simulink model "vegas01_4tap_1024ch_220_r8b"  (Simulink 7.6)
settings: Solver=ode45  StartTime=0.0  StopTime=128000  RelTol=1e-3
libraries: xbsIndex_r4 x12586, monroe_library x80, xbsReplacements_r4 x42, xps_library x34, casper_library_misc x27, dspsigops x16, simulink x7
(part 9 of 91)

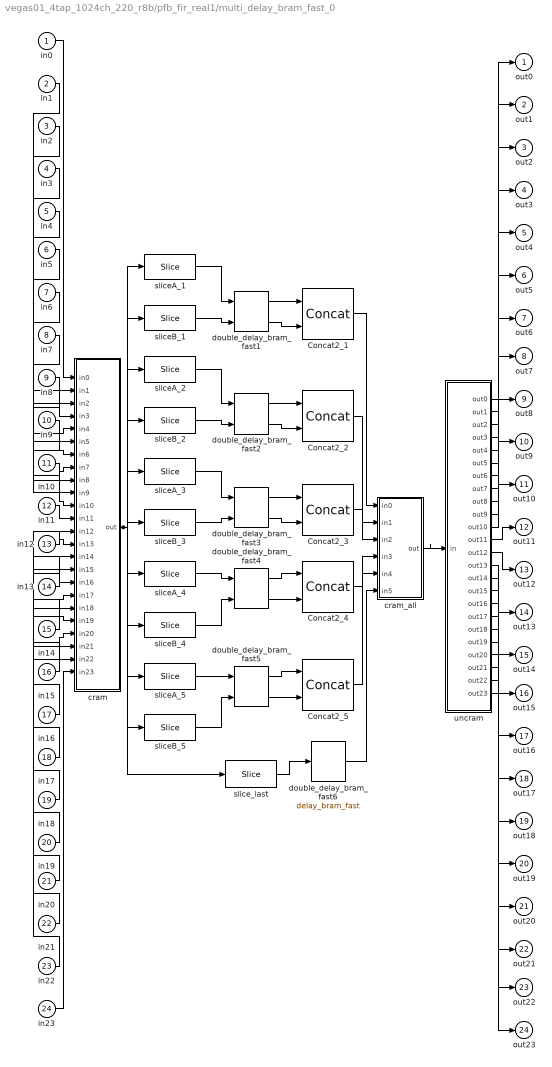
== system vegas01_4tap_1024ch_220_r8b/pfb_fir_real1/multi_delay_bram_fast_0 ==
  in0 : Inport
  in1 : Inport  Port=2
  in2 : Inport  Port=3
  in3 : Inport  Port=4
  in4 : Inport  Port=5
  in5 : Inport  Port=6
  in6 : Inport  Port=7
  in7 : Inport  Port=8
  in8 : Inport  Port=9
  in9 : Inport  Port=10
  in10 : Inport  Port=11
  in11 : Inport  Port=12
  in12 : Inport  Port=13
  in13 : Inport  Port=14
  in14 : Inport  Port=15
  in15 : Inport  Port=16
  in16 : Inport  Port=17
  in17 : Inport  Port=18
  in18 : Inport  Port=19
  in19 : Inport  Port=20
  in20 : Inport  Port=21
  in21 : Inport  Port=22
  in22 : Inport  Port=23
  in23 : Inport  Port=24
  Concat2_{1..5} (5 alike) : xbsIndex_r4/Concat  num_inputs=2  dbl_ovrd=off  block_type=concat
  cram : Subsystem
  cram_all : Subsystem
  double_delay_bram_fast{1..5} (5 alike) : monroe_library/double_delay_bram_fast  SystemSampleTime=-1  delay_len=256  bram_latency=2  register_counter=1
  double_delay_bram_fast6 : monroe_library/delay_bram_fast  SystemSampleTime=-1  delay_len=256  bram_latency=2  memory_type=Block RAM  register_counter=1
  sliceA_1 : xbsIndex_r4/Slice  nbits=18  boolean_output=off  mode=Upper Bit Location + Width  bit1=-0  base1=MSB of Input  bit0=-0  base0=MSB of Input  dbl_ovrd=off  block_type=slice
  sliceA_2 : xbsIndex_r4/Slice  nbits=18  boolean_output=off  mode=Upper Bit Location + Width  bit1=-36  base1=MSB of Input  bit0=-36  base0=MSB of Input  dbl_ovrd=off  block_type=slice
  sliceA_3 : xbsIndex_r4/Slice  nbits=18  boolean_output=off  mode=Upper Bit Location + Width  bit1=-72  base1=MSB of Input  bit0=-72  base0=MSB of Input  dbl_ovrd=off  block_type=slice
  sliceA_4 : xbsIndex_r4/Slice  nbits=18  boolean_output=off  mode=Upper Bit Location + Width  bit1=-108  base1=MSB of Input  bit0=-108  base0=MSB of Input  dbl_ovrd=off  block_type=slice
  sliceA_5 : xbsIndex_r4/Slice  nbits=18  boolean_output=off  mode=Upper Bit Location + Width  bit1=-144  base1=MSB of Input  bit0=-144  base0=MSB of Input  dbl_ovrd=off  block_type=slice
  sliceB_1 : xbsIndex_r4/Slice  nbits=18  boolean_output=off  mode=Upper Bit Location + Width  bit1=-18  base1=MSB of Input  bit0=-18  base0=MSB of Input  dbl_ovrd=off  block_type=slice
  sliceB_2 : xbsIndex_r4/Slice  nbits=18  boolean_output=off  mode=Upper Bit Location + Width  bit1=-54  base1=MSB of Input  bit0=-54  base0=MSB of Input  dbl_ovrd=off  block_type=slice
  sliceB_3 : xbsIndex_r4/Slice  nbits=18  boolean_output=off  mode=Upper Bit Location + Width  bit1=-90  base1=MSB of Input  bit0=-90  base0=MSB of Input  dbl_ovrd=off  block_type=slice
  sliceB_4 : xbsIndex_r4/Slice  nbits=18  boolean_output=off  mode=Upper Bit Location + Width  bit1=-126  base1=MSB of Input  bit0=-126  base0=MSB of Input  dbl_ovrd=off  block_type=slice
  sliceB_5 : xbsIndex_r4/Slice  nbits=18  boolean_output=off  mode=Upper Bit Location + Width  bit1=-162  base1=MSB of Input  bit0=-162  base0=MSB of Input  dbl_ovrd=off  block_type=slice
  slice_last : xbsIndex_r4/Slice  nbits=12  boolean_output=off  mode=Lower Bit Location + Width  bit1=0  base1=LSB of Input  bit0=0  base0=LSB of Input  dbl_ovrd=off  block_type=slice
  uncram : Subsystem
  out0 : Outport
  out1 : Outport  Port=2
  out2 : Outport  Port=3
  out3 : Outport  Port=4
  out4 : Outport  Port=5
  out5 : Outport  Port=6
  out6 : Outport  Port=7
  out7 : Outport  Port=8
  out8 : Outport  Port=9
  out9 : Outport  Port=10
  out10 : Outport  Port=11
  out11 : Outport  Port=12
  out12 : Outport  Port=13
  out13 : Outport  Port=14
  out14 : Outport  Port=15
  out15 : Outport  Port=16
  out16 : Outport  Port=17
  out17 : Outport  Port=18
  out18 : Outport  Port=19
  out19 : Outport  Port=20
  out20 : Outport  Port=21
  out21 : Outport  Port=22
  out22 : Outport  Port=23
  out23 : Outport  Port=24
  wires:
  cram/1(out) -> slice_last/1, sliceB_5/1, sliceA_5/1, sliceB_4/1, sliceA_4/1, sliceB_3/1, sliceA_3/1, sliceB_2/1, sliceA_2/1, sliceB_1/1, sliceA_1/1
  uncram/24(out23) -> out23/1
  uncram/23(out22) -> out22/1
  uncram/22(out21) -> out21/1
  uncram/21(out20) -> out20/1
  uncram/20(out19) -> out19/1
  uncram/19(out18) -> out18/1
  uncram/18(out17) -> out17/1
  uncram/17(out16) -> out16/1
  uncram/16(out15) -> out15/1
  uncram/15(out14) -> out14/1
  uncram/14(out13) -> out13/1
  uncram/13(out12) -> out12/1
  uncram/12(out11) -> out11/1
  uncram/11(out10) -> out10/1
  uncram/10(out9) -> out9/1
  uncram/9(out8) -> out8/1
  uncram/8(out7) -> out7/1
  uncram/7(out6) -> out6/1
  uncram/6(out5) -> out5/1
  uncram/5(out4) -> out4/1
  uncram/4(out3) -> out3/1
  uncram/3(out2) -> out2/1
  uncram/2(out1) -> out1/1
  uncram/1(out0) -> out0/1
  cram_all/1(out) -> uncram/1(in)
  double_delay_bram_fast6/1 -> cram_all/6(in5)
  Concat2_5/1 -> cram_all/5(in4)
  Concat2_4/1 -> cram_all/4(in3)
  Concat2_3/1 -> cram_all/3(in2)
  Concat2_2/1 -> cram_all/2(in1)
  Concat2_1/1 -> cram_all/1(in0)
  slice_last/1 -> double_delay_bram_fast6/1
  double_delay_bram_fast5/2 -> Concat2_5/2
  double_delay_bram_fast5/1 -> Concat2_5/1
  sliceB_5/1 -> double_delay_bram_fast5/2
  sliceA_5/1 -> double_delay_bram_fast5/1
  double_delay_bram_fast4/2 -> Concat2_4/2
  double_delay_bram_fast4/1 -> Concat2_4/1
  sliceB_4/1 -> double_delay_bram_fast4/2
  sliceA_4/1 -> double_delay_bram_fast4/1
  double_delay_bram_fast3/2 -> Concat2_3/2
  double_delay_bram_fast3/1 -> Concat2_3/1
  sliceB_3/1 -> double_delay_bram_fast3/2
  sliceA_3/1 -> double_delay_bram_fast3/1
  double_delay_bram_fast2/2 -> Concat2_2/2
  double_delay_bram_fast2/1 -> Concat2_2/1
  sliceB_2/1 -> double_delay_bram_fast2/2
  sliceA_2/1 -> double_delay_bram_fast2/1
  double_delay_bram_fast1/2 -> Concat2_1/2
  double_delay_bram_fast1/1 -> Concat2_1/1
  sliceB_1/1 -> double_delay_bram_fast1/2
  sliceA_1/1 -> double_delay_bram_fast1/1
  in23/1 -> cram/24(in23)
  in22/1 -> cram/23(in22)
  in21/1 -> cram/22(in21)
  in20/1 -> cram/21(in20)
  in19/1 -> cram/20(in19)
  in18/1 -> cram/19(in18)
  in17/1 -> cram/18(in17)
  in16/1 -> cram/17(in16)
  in15/1 -> cram/16(in15)
  in14/1 -> cram/15(in14)
  in13/1 -> cram/14(in13)
  in12/1 -> cram/13(in12)
  in11/1 -> cram/12(in11)
  in10/1 -> cram/11(in10)
  in9/1 -> cram/10(in9)
  in8/1 -> cram/9(in8)
  in7/1 -> cram/8(in7)
  in6/1 -> cram/7(in6)
  in5/1 -> cram/6(in5)
  in4/1 -> cram/5(in4)
  in3/1 -> cram/4(in3)
  in2/1 -> cram/3(in2)
  in1/1 -> cram/2(in1)
  in0/1 -> cram/1(in0)

== system vegas01_4tap_1024ch_220_r8b/pfb_fir_real3/coeff_gen_0_7 ==
  sync : Inport
  Counter0 : xbsIndex_r4/Counter  cnt_type=Free Running  cnt_to=Inf  operation=Up  start_count=8  cnt_by_val=1  arith_type=Unsigned  n_bits=8  bin_pt=0  load_pin=off  rst=on  en=off  explicit_period=on  period=1  dbl_ovrd=off  use_behavioral_HDL=off  implementation=Fabric  block_type=counter
  Counter1 : xbsIndex_r4/Counter  cnt_type=Free Running  cnt_to=Inf  operation=Down  start_count=247  cnt_by_val=1  arith_type=Unsigned  n_bits=8  bin_pt=0  load_pin=off  rst=on  en=off  explicit_period=on  period=1  dbl_ovrd=off  use_behavioral_HDL=off  implementation=Fabric  block_type=counter
  Delay1 : xbsIndex_r4/Delay  rst=off  infoeditControl=Selection of Reset will increase slice count due to use of real FFs and instead of SRLs  en=off  latency=2  dbl_ovrd=off  reg_retiming=off  block_type=delay
  Delay2 : xbsIndex_r4/Delay  rst=off  infoeditControl=Selection of Reset will increase slice count due to use of real FFs and instead of SRLs  en=off  latency=1  dbl_ovrd=off  reg_retiming=off  block_type=delay
  Delay3 : xbsIndex_r4/Delay  rst=off  infoeditControl=Selection of Reset will increase slice count due to use of real FFs and instead of SRLs  en=off  latency=1  dbl_ovrd=off  reg_retiming=off  block_type=delay
  Delay4 : xbsIndex_r4/Delay  rst=off  infoeditControl=Selection of Reset will increase slice count due to use of real FFs and instead of SRLs  en=off  latency=2  dbl_ovrd=off  reg_retiming=off  block_type=delay
  coeff_ram_1 : xbsIndex_r4/Dual Port RAM  depth=256  initVector=[-9.76681628083641e-06 -0.000166374153578663 -0.000323674631039594 -0.000481750704462836 -0.000640685368821483 -0.000800562109341241 -0.000961464852068701 -0.00112347791394463 -0.00128668595239865 -0.00145117391448125 -0.00161702698554985 -0.00178433053752527 -0.00195317007673584 -0.00212363119136582 -0.00229579949852553 -0.00246976059096078 -0.00264559998341877 -0.00282340305868871 -0.00300325501333463 -0.003185240803139 -0.00336944508827481 -0.00355595217822514 -0.00374484597646818 -0.00393620992494696 -0.0041301269483421 -0.00432667939816694 -0.00452594899670393 -0.00472801678080149 -0.00493296304555074 -0.00514086728786142 -0.00535180814995662 -0.0055658633628058 -0.00578310968951604 -0.00600362286870091 -0.00622747755784734 -0.00645474727669994 -0.00668550435068305 -0.00691981985438059 -0.00715776355509352 -0.00739940385649554 -0.00764480774240668 -0.00789404072070559 -0.00814716676740016 -0.00840424827087738 -0.00866534597635216 -0.00893051893053586 -0.00919982442654445 -0.00947331794906695 -0.00975105311981421 -0.0100330816432682 -0.0103194532527527 -0.010610215656844 -0.0109054144861447 -0.0112050932404367 -0.011509293236238 -0.0118180535547789 -0.0121314109904214 -0.0124493999995389 -0.0127720526498779 -0.0130993985704197 -0.0134314649017638 -0.0137682762470507 -0.0141098546234445 -0.0144562194141947 -0.0148073873212959 -0.0151633723187648 -0.015524185606554 -0.0158898355651202 -0.0162603277106669 -0.0166356646510793 -0.0170158460425702 -0.0174008685470547 -0.0177907257902727 -0.0181854083206757 -0.0185849035690979 -0.0189891958092265 -0.0193982661188903 -0.0198120923421836 -0.0202306490524425 -0.0206539075160887 -0.0210818356573608 -0.0215143980239454 -0.0219515557535275 -0.0223932665412744 -0.0228394846082689 -0.0232901606709079 -0.0237452419112808 -0.0242046719485435 -0.0246683908113017 -0.0251363349110189 -0.0256084370164626 -0.0260846262292031 -0.0265648279601778 -0.0270489639073352 -0.0275369520343712 -0.0280287065505702 -0.0285241378917642 -0.0290231527024214 -0.0295256538188765 -0.0300315402537142 -0.0305407071813169 -0.0310530459245885 -0.0315684439428629 -0.0320867848210099 -0.0326079482597467 -0.0331318100671657 -0.0336582421514863 -0.0341871125150421 -0.0347182852495099 -0.0352516205323896 -0.0357869746247431 -0.0363241998701992 -0.0368631446952324 -0.0374036536107213 -0.0379455672147943 -0.0384887221969679 -0.0390329513435835 -0.0395780835445478 -0.0401239438013823 -0.0406703532365864 -0.0412171291043174 -0.0417640848023921 -0.0423110298856138 -0.0428577700804262 -0.0434041073008984 -0.0439498396660427 -0.0444947615184662 -0.0450386634443597 -0.0455813322948223 -0.0461225512085252 -0.0466620996357123 -0.0471997533635396 -0.0477352845427513 -0.0482684617156931 -0.0487990498456597 -0.0493268103475761 -0.0498515011200101 -0.0503728765785135 -0.0508906876902881 -0.0514046820101748 -0.0519146037179613 -0.0524201936570026 -0.0529211893741533 -0.0534173251610023 -0.0539083320964084 -0.0543939380903286 -0.0548738679289332 -0.0553478433210022 -0.0558155829455944 -0.0562768025009831 -0.0567312147548497 -0.0571785295957271 -0.0576184540856848 -0.0580506925142456 -0.0584749464535256 -0.0588909148145869 -0.0592982939049929 -0.0596967774875561 -0.0600860568402664 -0.0604658208173894 -0.0608357559117222 -0.0611955463179954 -0.0615448739974078 -0.0618834187432817 -0.0622108582478242 -0.062526868169983 -0.0628311222043803 -0.0631232921513118 -0.0634030479877959 -0.0636700579396572 -0.0639239885546298 -0.0641645047764638 -0.0643912700200192 -0.0646039462473303 -0.0648021940446247 -0.0649856727002773 -0.0651540402836856 -0.065306953725044 -0.0654440688960025 -0.0655650406911897 -0.06566952311058 -0.0657571693426894 -0.0658276318485765 -0.0658805624466314 -0.0659156123981315 -0.0659324324935443 -0.0659306731395561 -0.0659099844468056 -0.0658700163183022 -0.0658104185385061 -0.0657308408630492 -0.065630933109075 -0.0655103452461749 -0.0653687274878981 -0.0652057303838135 -0.0650210049120985 -0.0648142025726343 ...(+1180 chars)]  distributed_mem=Block RAM  init_a=0  init_b=0  rst_a=off  rst_b=off  en_a=off  en_b=off  latency=2  write_mode_A=No Read On Write  write_mode_B=No Read On Write  dbl_ovrd=off  optimize=Area  use_rpm=on  block_type=dpram
  coeff_ram_2 : xbsIndex_r4/Dual Port RAM  depth=256  initVector=[0.000131889660979235 0.0022626199016281 0.00443201888730544 0.00664021076774132 0.00888731333319452 0.011173437943922 0.013498689460842 0.0158631661774126 0.0182669597527502 0.0207101551460066 0.0231928305520307 0.0257150573383326 0.0282768999833737 0.0308784160162031 0.0335196559574605 0.0362006632617669 0.0389214742615229 0.041682118112133 0.0444826167386781 0.0473229847840524 0.0502032295585852 0.0531233509911659 0.0560833415818892 0.0590831863562395 0.0621228628208312 0.0652023409207219 0.0683215829983153 0.07148054375387 0.0746791702076299 0.0779174016635925 0.0811951696749281 0.084512398011068 0.0878690026264726 0.0912648916310948 0.0946999652625533 0.0981741158600261 0.10168722783988 0.105239177673046 0.108829833864151 0.112459056932427 0.116126699394389 0.119832605748316 0.123576612460526 0.127358547953464 0.131178232595614 0.135035478693229 0.138930090483912 0.142861864132034 0.146830587726002 0.150836041277392 0.154877996721945 0.158956217922433 0.163070460673406 0.167220472707813 0.171405993705518 0.175626755303703 0.179882481109162 0.184172886712494 0.188497679704198 0.192856559692668 0.197249218324085 0.201675339304225 0.206134598422161 0.210626663575877 0.215151194799783 0.219707844294133 0.224296256456348 0.228916067914241 0.233566907561132 0.238248396592867 0.242960148546725 0.247701769342212 0.252472857323742 0.257273003305192 0.262101790616336 0.266958795151142 0.271843585417932 0.276755722591397 0.28169476056646 0.286660246013975 0.291651718438258 0.296668710236444 0.301710746759654 0.306777346375963 0.311868020535164 0.316982273835315 0.322119604091054 0.327279502403677 0.332461453232956 0.337664934470704 0.342889417516048 0.348134367352417 0.353399242626224 0.358683495727226 0.363986572870548 0.369307914180353 0.374646953775156 0.380003119854737 0.385375834788674 0.390764515206442 0.396168572089086 0.401587410862443 0.407020431491882 0.412467028578566 0.417926591457195 0.423398504295229 0.428882146193547 0.434376891288555 0.439882108855684 0.445397163414288 0.450921414833902 0.456454218441849 0.461994925132158 0.467542881475792 0.473097429832141 0.478657908461773 0.48422365164041 0.489793989774102 0.495368249515587 0.500945753881801 0.506525822372513 0.512107771090063 0.517690912860181 0.523274557353848 0.528858011210183 0.534440578160332 0.540021559152312 0.545600252476811 0.551175953893889 0.556747956760575 0.562315552159312 0.567878029027235 0.573434674286248 0.578984772973868 0.584527608374814 0.590062462153306 0.595588614486041 0.601105344195828 0.606611928885839 0.61210764507445 0.617591768330647 0.623063573409952 0.628522334390862 0.633967324811734 0.639397817808129 0.644813086250539 0.650212402882503 0.655595040459053 0.660960271885469 0.666307370356321 0.671635609494744 0.676944263491929 0.682232607246797 0.687499916505816 0.692745468002935 0.697968539599602 0.703168410424828 0.708344361015273 0.713495673455308 0.718621631517039 0.723721520800239 0.728794628872177 0.733840245407289 0.738857662326676 0.743846173937386 0.748805077071453 0.753733671224655 0.758631258694962 0.763497144720641 0.768330637617979 0.773131048918607 0.77789769350637 0.782629889753732 0.787326959657669 0.791988228975025 0.796613027357295 0.801200688484807 0.805750550200266 0.810261954641631 0.814734248374296 0.819166782522532 0.823558912900179 0.827910000140534 0.832219409825417 0.836486512613386 0.840710684367061 0.844891306279528 0.849027764999809 0.853119452757334 0.857165767485426 0.861166112943733 0.865119898839605 0.869026540948364 0.872885461232459 0.876696087959463 0.880457855818883 0.884170206037768 0.887832586495072 0.891444451834754 0.895005263577584 0.898514490231627 0.90197160740138 0.905376097895536 0.908727451833345 0.912025166749558 0.915268747697902 0.9184577073531 0.921591566111373 0.924669852189424 0.927692101721869 0.930657858857099 0.933566675851536 0.936418113162282 0.939211739538114 0.941947132108819 0.944623876472843 0.947241566783232 0.949799805831843 0.952298205131806 0.954736384998219 ...(+644 chars)]  distributed_mem=Block RAM  init_a=0  init_b=0  rst_a=off  rst_b=off  en_a=off  en_b=off  latency=2  write_mode_A=No Read On Write  write_mode_B=No Read On Write  dbl_ovrd=off  optimize=Area  use_rpm=on  block_type=dpram
  coeff_ram_3 : xbsIndex_r4/Dual Port RAM  depth=256  initVector=[0.999999868120266 0.999961887307505 0.999856390808123 0.999683394879622 0.999442926180455 0.999135021764442 0.998759729072997 0.99831710592518 0.997807220505575 0.997230151349991 0.996585987329003 0.995874827629316 0.995096781732979 0.994251969394439 0.993340520615446 0.992362575617817 0.99131828481406 0.99020780877587 0.989031318200503 0.98778899387504 0.986481026638539 0.985107617342099 0.983668976806834 0.982165325779773 0.980596894887697 0.978963924588919 0.977266665123027 0.975505376458598 0.973680328238889 0.971791799725535 0.969840079740251 0.967825466604565 0.96574826807758 0.963608801291808 0.961407392687059 0.959144377942428 0.956820101906379 0.954434918524957 0.95198919076813 0.949483290554292 0.946917598672946 0.94429250470557 0.941608406944702 0.938865712311265 0.936064836270128 0.933206202743959 0.930290244025361 0.927317400687328 0.924288121492039 0.921202863298015 0.918062090965654 0.914866277261172 0.91161590275898 0.90831145574251 0.904953432103512 0.901542335239865 0.898078675951906 0.894562972337311 0.890995749684561 0.887377540364997 0.883708883723521 0.879990325967942 0.876222420057003 0.872405725587129 0.868540808677901 0.864628241856301 0.860668603939747 0.856662479917952 0.852610460833631 0.848513143662084 0.844371131189694 0.840185031891353 0.835955459806863 0.831683034416324 0.827368380514556 0.823012128084576 0.818614912170158 0.814177372747513 0.809700154596117 0.805183907168721 0.800629284460566 0.79603694487784 0.791407551105419 0.786741769973893 0.782040272325951 0.777303732882114 0.772532830105885 0.767728246068318 0.762890666312066 0.758020779714911 0.753119278352835 0.748186857362649 0.743224214804217 0.738232051522307 0.733211071008104 0.728161979260409 0.723085484646579 0.717982297763205 0.712853131296598 0.707698699883091 0.702519719969201 0.697316909671679 0.692090988637482 0.686842677903704 0.681572699757496 0.676281777595997 0.670970635786334 0.665639999525697 0.660290594701535 0.65492314775191 0.649538385526024 0.644137035144977 0.638719823862754 0.633287478927515 0.627840727443177 0.622380296231356 0.616906911693676 0.611421299674493 0.605924185324051 0.600416292962115 0.5948983459421 0.589371066515746 0.583835175698334 0.578291393134523 0.572740436964789 0.567183023692534 0.561619868051874 0.556051682876141 0.550479178967135 0.544903064965141 0.539324047219761 0.533742829661563 0.528160113674606 0.522576597969841 0.516992978459435 0.511409948132039 0.50582819692903 0.500248411621744 0.494671275689747 0.489097469200151 0.48352766868801 0.477962547037825 0.472402773366174 0.466849012905507 0.461301926889109 0.455762172437285 0.45023040244476 0.444707265469343 0.439193405621868 0.433689462457427 0.428196070867941 0.422713860976064 0.417243458030464 0.411785482302493 0.406340548984262 0.400909268088159 0.39549224434781 0.390090077120521 0.384703360291205 0.379332682177829 0.373978625438387 0.36864176697942 0.363322677866115 0.358021923233973 0.35274006220209 0.347477647788056 0.342235226824484 0.337013339877193 0.331812521165061 0.32663329848155 0.321476193117935 0.316341719788239 0.311230386555891 0.306142694762123 0.301079138956108 0.296040206826872 0.291026379136963 0.286038129657917 0.281075925107508 0.276140225088811 0.271231482031073 0.266350141132408 0.26149664030433 0.256671410118117 0.25187487375303 0.247107446946385 0.24236953794549 0.237661547461441 0.232983868624808 0.228336886943181 0.223720980260621 0.21913651871898 0.214583864721127 0.210063372896065 0.205575390065943 0.201120255214974 0.196698299460255 0.192309846024486 0.187955210210599 0.183634699378287 0.179348612922444 0.175097242253503 0.170880870779681 0.166699773891124 0.162554218945948 0.158444465258183 0.154370764087607 0.150333358631466 0.146332484018087 0.142368367302369 0.138441227463148 0.134551275402435 0.130698713946519 0.126883737848928 0.123106533795237 0.119367280409732 0.115666148263892 0.112003299886717 0.108378889776859 0.104793064416578 0.101245962287486 0.0977377138880848 0.0942684417530879 ...(+629 chars)]  distributed_mem=Block RAM  init_a=0  init_b=0  rst_a=off  rst_b=off  en_a=off  en_b=off  latency=2  write_mode_A=No Read On Write  write_mode_B=No Read On Write  dbl_ovrd=off  optimize=Area  use_rpm=on  block_type=dpram
  coeff_ram_4 : xbsIndex_r4/Dual Port RAM  depth=256  initVector=[-0.000131739151355809 -0.00221912327775564 -0.00426812326391606 -0.00627888266556972 -0.00825155109486444 -0.0101862841440803 -0.0120832433082974 -0.0139425959070377 -0.0157645150049071 -0.0175491793312596 -0.0192967731989124 -0.0210074864219326 -0.0226815142325243 -0.0243190571970391 -0.0259203211311373 -0.0274855170141237 -0.0290148609024857 -0.0305085738426582 -0.0319668817830422 -0.0333900154853026 -0.0347782104349725 -0.0361317067513902 -0.0374507490969939 -0.0387355865860033 -0.0399864726925128 -0.0412036651580236 -0.0423874258984425 -0.0435380209105723 -0.044655720178123 -0.0457407975772691 -0.0467935307817807 -0.0478142011677561 -0.0488030937179814 -0.0497604969259452 -0.0506867026995366 -0.0515820062644503 -0.0524467060673303 -0.0532811036786751 -0.0540855036955342 -0.0548602136440219 -0.0556055438816755 -0.0563218074996844 -0.0570093202250184 -0.0576684003224797 -0.0582993684967072 -0.05890254779416 -0.0594782635051042 -0.0600268430656328 -0.0605486159597422 -0.0610439136214937 -0.0615130693372847 -0.0619564181482566 -0.0623742967528647 -0.0627670434096362 -0.0631349978401424 -0.0634785011322087 -0.0637978956433909 -0.06409352490474 -0.0643657335248817 -0.0646148670944361 -0.064841272090801 -0.0650452957833243 -0.0652272861388886 -0.0653875917279336 -0.0655265616309388 -0.0656445453453909 -0.0657418926932587 -0.0658189537289998 -0.0658760786481207 -0.0659136176963139 -0.0659319210791943 -0.065931338872657 -0.0659122209338789 -0.0658749168129847 -0.0658197756654005 -0.0657471461649141 -0.0656573764174654 -0.0655508138756844 -0.0654278052542005 -0.0652886964457401 -0.0651338324380346 -0.0649635572315565 -0.0647782137581042 -0.0645781438002524 -0.0643636879116891 -0.0641351853384555 -0.0638929739411062 -0.0636373901178102 -0.063368768728406 -0.0630874430194303 -0.0627937445501364 -0.0624880031195173 -0.0621705466943507 -0.0618417013382798 -0.0615017911419477 -0.061151138154196 -0.0607900623143467 -0.060418881385577 -0.0600379108894042 -0.0596474640412915 -0.0592478516873886 -0.0588393822424205 -0.058422361628734 -0.0579970932165166 -0.0575638777651976 -0.057123013366042 -0.0566747953859491 -0.056219516412465 -0.055757466200019 -0.0552889316173935 -0.0548141965964363 -0.0543335420820237 -0.0538472459832827 -0.0533555831260807 -0.0528588252067891 -0.0523572407473282 -0.0518510950515002 -0.0513406501626167 -0.0508261648224251 -0.0503078944313416 -0.0497860910099933 -0.0492610031620756 -0.0487328760385286 -0.0482019513030358 -0.0476684670988495 -0.0471326580169441 -0.0465947550655016 -0.0460549856407311 -0.0455135734990219 -0.044970738730435 -0.0444266977335309 -0.0438816631915359 -0.0433358440498461 -0.0427894454948693 -0.0422426689342039 -0.0416957119781535 -0.0411487684225764 -0.0406020282330669 -0.0400556775304669 -0.0395098985777058 -0.0389648697679635 -0.0384207656141553 -0.0378777567397334 -0.0373360098708014 -0.0367956878295371 -0.036256949528918 -0.035719949968745 -0.0351848402329585 -0.0346517674882394 -0.0341208749838907 -0.0335923020529909 -0.0330661841148128 -0.0325426526785005 -0.0320218353479964 -0.0315038558282083 -0.0309888339324118 -0.0304768855908741 -0.0299681228606948 -0.0294626539368496 -0.0289605831644304 -0.0284620110520695 -0.0279670342865377 -0.0274757457485057 -0.0269882345294571 -0.0265045859497419 -0.0260248815777572 -0.0255491992502456 -0.0250776130936957 -0.0246101935468339 -0.0241470073841942 -0.0236881177407513 -0.0232335841376052 -0.0227834625087025 -0.0223378052285791 -0.0218966611411123 -0.0214600755892652 -0.02102809044581 -0.0206007441450138 -0.0201780717152727 -0.0197601048126773 -0.0193468717554942 -0.0189383975595475 -0.0185347039744836 -0.0181358095209027 -0.0177417295283403 -0.0173524761740819 -0.016968058522793 -0.0165884825669475 -0.0162137512680375 -0.0158438645985451 -0.0154788195846594 -0.0151186103497212 -0.0147632281583751 -0.0144126614614124 -0.0140668959412854 -0.0137259145582748 -0.0133896975972904 -0.0130582227152872 -0.0127314649892776 -0.0124093969649192 ...(+1171 chars)]  distributed_mem=Block RAM  init_a=0  init_b=0  rst_a=off  rst_b=off  en_a=off  en_b=off  latency=2  write_mode_A=No Read On Write  write_mode_B=No Read On Write  dbl_ovrd=off  optimize=Area  use_rpm=on  block_type=dpram
  const_din : xbsIndex_r4/Constant  const=0  gui_display_data_type=Fixed-point  arith_type=Signed (2's comp)  n_bits=18  bin_pt=17  preci_type=Single  exp_width=8  frac_width=24  explicit_period=on  period=1  equ=P=C  opselect=C  inp2=PCIN>>17  opr=+  inp1=P  carry=CIN  dbl_ovrd=off  block_type=constant
  const_we : xbsIndex_r4/Constant  const=0  gui_display_data_type=Fixed-point  arith_type=Boolean  n_bits=16  bin_pt=14  preci_type=Single  exp_width=8  frac_width=24  explicit_period=on  period=1  equ=P=C  opselect=C  inp2=PCIN>>17  opr=+  inp1=P  carry=CIN  dbl_ovrd=off  block_type=constant
  cram_lower : Subsystem
  cram_upper : Subsystem
  sync_out : Outport
  coeff : Outport  Port=2
  coeff_rev : Outport  Port=3
  wires:
  sync/1 -> Counter1/1, Counter0/1, sync_out/1
  Counter0/1 -> coeff_ram_4/1, coeff_ram_3/1, Delay2/1, Delay1/1
  const_din/1 -> coeff_ram_4/5, coeff_ram_4/2, coeff_ram_3/5, coeff_ram_3/2, coeff_ram_2/5, coeff_ram_2/2, coeff_ram_1/5, coeff_ram_1/2
  const_we/1 -> coeff_ram_4/6, coeff_ram_4/3, coeff_ram_3/6, coeff_ram_3/3, coeff_ram_2/6, coeff_ram_2/3, coeff_ram_1/6, coeff_ram_1/3
  Counter1/1 -> Delay4/1, Delay3/1, coeff_ram_2/4, coeff_ram_1/4
  cram_lower/1(out) -> coeff_rev/1
  cram_upper/1(out) -> coeff/1
  coeff_ram_1/2 -> cram_lower/4(in3)
  coeff_ram_2/2 -> cram_lower/3(in2)
  coeff_ram_3/2 -> cram_lower/2(in1)
  coeff_ram_4/2 -> cram_lower/1(in0)
  coeff_ram_4/1 -> cram_upper/4(in3)
  coeff_ram_3/1 -> cram_upper/3(in2)
  coeff_ram_2/1 -> cram_upper/2(in1)
  coeff_ram_1/1 -> cram_upper/1(in0)
  Delay4/1 -> coeff_ram_4/4
  Delay3/1 -> coeff_ram_3/4
  Delay2/1 -> coeff_ram_2/1
  Delay1/1 -> coeff_ram_1/1
